simulink model "AcasXuClosedLoop_all_comp2017b"  (Simulink 9.0)
settings: Solver=VariableStepAuto  StartTime=0.0  StopTime=20  RelTol=1e-3
libraries: neural x14040, simulink x2
(part 24 of 534)

== system AcasXuClosedLoop_all_comp2017b/networks/Networks_m_5/AcasXu_5_5/Layer 3 ==
  "a{2} " : Inport  PortDimensions=50
      mask Display: e = 0.06;c1 = [120 230 180]/255;c2 = ([120 230 180]+0)/(256+128);c3 = ([120 230 180]+128)/(256+128);patch([0 1 1 0],[0 0 1 1],c1);patch([0 1 1 1-e 1-e 0+e],[0 0 1 1-e 0+e 0+e],c2);patch([0 0 1 1-e 0+e 0+e],[0 1 1 1-e 1-e 0+e],c3);plot([0 1 1 0 0],[0 0 1 1 0]);
  Delays 1 : Subsystem
      mask Display: e = 0.06;c1 = [120 150 230]/255;c2 = ([120 150 230]+0)/383;c3 = ([120 150 230]+128)/383;patch([0 1 1 0],[0 0 1 1],c1);patch([0 1 1 1-e 1-e 0+e],[0 0 1 1-e 0+e 0+e],c2);patch([0 0 1 1-e 0+e 0+e],[0 1 1 1-e 1-e 0+e],c3);plot([0 1 1 0 0],[0 0 1 1 0]);disp('0');
  "LW{3,2}" : Subsystem
      mask Display: e = 0.06;c1 = [120 150 230]/255;c2 = ([120 150 230]+0)/383;c3 = ([120 150 230]+128)/383;patch([0 1 1 0],[0 0 1 1],c1);patch([0 1 1 1-e 1-e 0+e],[0 0 1 1-e 0+e 0+e],c2);patch([0 0 1 1-e 0+e 0+e],[0 1 1 1-e 1-e 0+e],c3);plot([0 1 1 0 0],[0 0 1 1 0]);disp('W');
  "b{3}" : Constant  Value=[0.7179569999999999563300434601842425763607025146484375;0.73998500000000000387245790989254601299762725830078125;0.450612999999999985778487143761594779789447784423828125;-0.284893999999999980587972459034062922000885009765625;-0.6379829999999999667892325305729173123836517333984375;-0.0190351999999999986490362147151245153509080410003662109375;-0.7305260000000000086828322309884242713451385498046875;-0.134921000000000013141487897883052937686443328857421875;-1.0406800000000000494537744089029729366302490234375;-0.497483999999999981778131541432230733335018157958984375;-1.8671699999999999963762320476234890520572662353515625;-0.431711000000000011400658195270807482302188873291015625;-1.249049999999999993605115378159098327159881591796875;-0.52125699999999997036326249144622124731540679931640625;-0.38881500000000002170708057747106067836284637451171875;-0.230169000000000012473577726268558762967586517333984375;0.2886290000000000244284592554322443902492523193359375;1.158020000000000049311665861750952899456024169921875;0.62621400000000004837374945054762065410614013671875;-0.435367999999999977234210746246390044689178466796875;-0.09715310000000000612008221878568292595446109771728515625;-1.7334400000000000918731757337809540331363677978515625;-0.032423699999999999743494072390603832900524139404296875;0.6112830000000000207904804483405314385890960693359375;0.0296526000000000011791456699938862584531307220458984375;0.806359000000000047947423809091560542583465576171875;0.89186799999999999410960072054876945912837982177734375;-0.352733000000000018747670083030243404209613800048828125;0.77193500000000003780087354243732988834381103515625;0.06677470000000000627249363560622441582381725311279296875;-0.08103789999999999604796130370232276618480682373046875;-0.39701100000000000278532752417959272861480712890625;-0.5575200000000000155608859131461940705776214599609375;-0.00284884999999999999342747969421907328069210052490234375;0.16597600000000001241318159372895024716854095458984375;-0.289582999999999979312548248344683088362216949462890625;-0.346407999999999993701038647486711852252483367919921875;-0.174549000000000009702461056804168038070201873779296875;-0.771900000000000030553337637684307992458343505859375;0.35227799999999998004085455249878577888011932373046875;-0.704188999999999953871565594454295933246612548828125;-0.73722100000000001518429826319334097206592559814453125;-0.037192599999999999271782513687867322005331516265869140625;0.902943000000000051130655265296809375286102294921875;-0.25704300000000002146549604731262661516666412353515625;1.3462799999999999212008106042048893868923187255859375;-0.07558220000000000216022755239464459009468555450439453125;-0.04270920000000000271711542154662311077117919921875;-1.5134700000000000930100441109971143305301666259765625;0.58542000000000005144329406903125345706939697265625]
      mask Display: e = 0.06;c1 = [120 150 230]/255;c2 = ([120 150 230]+0)/383;c3 = ([120 150 230]+128)/383;patch([0 1 1 0],[0 0 1 1],c1);patch([0 1 1 1-e 1-e 0+e],[0 0 1 1-e 0+e 0+e],c2);patch([0 0 1 1-e 0+e 0+e],[0 1 1 1-e 1-e 0+e],c3);plot([0 1 1 0 0],[0 0 1 1 0]);disp('b');
  netsum : Sum  Inputs=2  SaturateOnIntegerOverflow=on  InputSameDT=on  OutDataTypeStr=Inherit: Same as first input
      mask Display: e = 0.06;c1 = [120 150 230]/255;c2 = ([120 150 230]+0)/383;c3 = ([120 150 230]+128)/383;patch([0 1 1 0],[0 0 1 1],c1);patch([0 1 1 1-e 1-e 0+e],[0 0 1 1-e 0+e 0+e],c2);patch([0 0 1 1-e 0+e 0+e],[0 1 1 1-e 1-e 0+e],c3);plot([0 1 1 0 0],[0 0 1 1 0]);disp('+');
  poslin : neural/Transfer Functions/poslin
      settings: SourceProductName=Deep Learning Toolbox  SourceProductBaseCode=NN
  "a{3}" : Outport  InitialOutput=[0;0;0;0;0;0;0;0;0;0;0;0;0;0;0;0;0;0;0;0;0;0;0;0;0;0;0;0;0;0;0;0;0;0;0;0;0;0;0;0;0;0;0;0;0;0;0;0;0;0]
      mask Display: e = 0.06;c1 = [120 230 180]/255;c2 = ([120 230 180]+0)/(256+128);c3 = ([120 230 180]+128)/(256+128);patch([0 1 1 0],[0 0 1 1],c1);patch([0 1 1 1-e 1-e 0+e],[0 0 1 1-e 0+e 0+e],c2);patch([0 0 1 1-e 0+e 0+e],[0 1 1 1-e 1-e 0+e],c3);plot([0 1 1 0 0],[0 0 1 1 0]);
  wires:
  poslin/1 -> "a{3}"/1
  netsum/1 -> poslin/1
  "b{3}"/1 -> netsum/2
  "LW{3,2}"/1("lz{3,2}") -> netsum/1
  Delays 1/1("pd{3,2}") -> "LW{3,2}"/1("ad{3,2}")
  "a{2} "/1 -> Delays 1/1("p{3}")

== system AcasXuClosedLoop_all_comp2017b/networks/Networks_m_5/AcasXu_5_5/Layer 4 ==
  "a{3} " : Inport  PortDimensions=50
      mask Display: e = 0.06;c1 = [120 230 180]/255;c2 = ([120 230 180]+0)/(256+128);c3 = ([120 230 180]+128)/(256+128);patch([0 1 1 0],[0 0 1 1],c1);patch([0 1 1 1-e 1-e 0+e],[0 0 1 1-e 0+e 0+e],c2);patch([0 0 1 1-e 0+e 0+e],[0 1 1 1-e 1-e 0+e],c3);plot([0 1 1 0 0],[0 0 1 1 0]);
  Delays 1 : Subsystem
      mask Display: e = 0.06;c1 = [120 150 230]/255;c2 = ([120 150 230]+0)/383;c3 = ([120 150 230]+128)/383;patch([0 1 1 0],[0 0 1 1],c1);patch([0 1 1 1-e 1-e 0+e],[0 0 1 1-e 0+e 0+e],c2);patch([0 0 1 1-e 0+e 0+e],[0 1 1 1-e 1-e 0+e],c3);plot([0 1 1 0 0],[0 0 1 1 0]);disp('0');
  "LW{4,3}" : Subsystem
      mask Display: e = 0.06;c1 = [120 150 230]/255;c2 = ([120 150 230]+0)/383;c3 = ([120 150 230]+128)/383;patch([0 1 1 0],[0 0 1 1],c1);patch([0 1 1 1-e 1-e 0+e],[0 0 1 1-e 0+e 0+e],c2);patch([0 0 1 1-e 0+e 0+e],[0 1 1 1-e 1-e 0+e],c3);plot([0 1 1 0 0],[0 0 1 1 0]);disp('W');
  "b{4}" : Constant  Value=[0.86561399999999999455013721672003157436847686767578125;-0.84666900000000000492406115881749428808689117431640625;0.0750065000000000037250202922223252244293689727783203125;0.283154999999999990034638130964594893157482147216796875;-4.12460999999999966547648000414483249187469482421875;0.082238699999999997913846527808345854282379150390625;-0.9694540000000000379287712348741479218006134033203125;0.687108999999999969787722875480540096759796142578125;0.515031000000000016569856597925536334514617919921875;-1.0304500000000000881072992342524230480194091796875;-0.038258699999999999652455784371340996585786342620849609375;-1.065770000000000106155084722558967769145965576171875;-2.813629999999999853343979339115321636199951171875;-1.8924099999999999255351212923415005207061767578125;-0.7152680000000000148929757415316998958587646484375;1.711580000000000101323394119390286505222320556640625;-0.619380000000000041637804315541870892047882080078125;-0.5122079999999999966320274324971251189708709716796875;-0.0881904000000000021231016944511793553829193115234375;-0.92888999999999999346300683100707828998565673828125;-0.01584549999999999847499765337488497607409954071044921875;0.50446100000000004826006261282600462436676025390625;-0.042949099999999997001598472934347228147089481353759765625;0.050072899999999996578647909473147592507302761077880859375;-0.75351299999999998835420456089195795357227325439453125;0.62020200000000003104361212535877712070941925048828125;0.686549999999999993605115378159098327159881591796875;2.18803999999999998493649400188587605953216552734375;0.320101000000000024403590259680640883743762969970703125;-2.126560000000000005826450433232821524143218994140625;-2.233659999999999978825826474349014461040496826171875;-0.484528000000000014235723710953607223927974700927734375;0.6396100000000000118660636871936731040477752685546875;1.960879999999999956372676024329848587512969970703125;2.0860500000000001818989403545856475830078125;-2.430330000000000101323394119390286505222320556640625;-1.0909299999999999553068619206896983087062835693359375;-2.556340000000000056701310313655994832515716552734375;-0.1785799999999999887467794223994133062660694122314453125;-0.57670600000000005191935770199052058160305023193359375;4.5265500000000002955857780762016773223876953125;-1.1713299999999999823074858795735053718090057373046875;0.133823999999999998511412968582590110599994659423828125;0.18771999999999999797495320308371447026729583740234375;-0.2106599999999999861532984368750476278364658355712890625;2.045310000000000183462134373257867991924285888671875;-0.72368299999999996519051137511269189417362213134765625;-2.34640000000000004121147867408581078052520751953125;0.553835999999999994969357430818490684032440185546875;-4.1325599999999997891109160264022648334503173828125]
      mask Display: e = 0.06;c1 = [120 150 230]/255;c2 = ([120 150 230]+0)/383;c3 = ([120 150 230]+128)/383;patch([0 1 1 0],[0 0 1 1],c1);patch([0 1 1 1-e 1-e 0+e],[0 0 1 1-e 0+e 0+e],c2);patch([0 0 1 1-e 0+e 0+e],[0 1 1 1-e 1-e 0+e],c3);plot([0 1 1 0 0],[0 0 1 1 0]);disp('b');
  netsum : Sum  Inputs=2  SaturateOnIntegerOverflow=on  InputSameDT=on  OutDataTypeStr=Inherit: Same as first input
      mask Display: e = 0.06;c1 = [120 150 230]/255;c2 = ([120 150 230]+0)/383;c3 = ([120 150 230]+128)/383;patch([0 1 1 0],[0 0 1 1],c1);patch([0 1 1 1-e 1-e 0+e],[0 0 1 1-e 0+e 0+e],c2);patch([0 0 1 1-e 0+e 0+e],[0 1 1 1-e 1-e 0+e],c3);plot([0 1 1 0 0],[0 0 1 1 0]);disp('+');
  poslin : neural/Transfer Functions/poslin
      settings: SourceProductName=Deep Learning Toolbox  SourceProductBaseCode=NN
  "a{4}" : Outport  InitialOutput=[0;0;0;0;0;0;0;0;0;0;0;0;0;0;0;0;0;0;0;0;0;0;0;0;0;0;0;0;0;0;0;0;0;0;0;0;0;0;0;0;0;0;0;0;0;0;0;0;0;0]
      mask Display: e = 0.06;c1 = [120 230 180]/255;c2 = ([120 230 180]+0)/(256+128);c3 = ([120 230 180]+128)/(256+128);patch([0 1 1 0],[0 0 1 1],c1);patch([0 1 1 1-e 1-e 0+e],[0 0 1 1-e 0+e 0+e],c2);patch([0 0 1 1-e 0+e 0+e],[0 1 1 1-e 1-e 0+e],c3);plot([0 1 1 0 0],[0 0 1 1 0]);
  wires:
  poslin/1 -> "a{4}"/1
  netsum/1 -> poslin/1
  "b{4}"/1 -> netsum/2
  "LW{4,3}"/1("lz{4,3}") -> netsum/1
  Delays 1/1("pd{4,3}") -> "LW{4,3}"/1("ad{4,3}")
  "a{3} "/1 -> Delays 1/1("p{4}")

== system AcasXuClosedLoop_all_comp2017b/networks/Networks_m_5/AcasXu_5_5/Layer 5 ==
  "a{4} " : Inport  PortDimensions=50
      mask Display: e = 0.06;c1 = [120 230 180]/255;c2 = ([120 230 180]+0)/(256+128);c3 = ([120 230 180]+128)/(256+128);patch([0 1 1 0],[0 0 1 1],c1);patch([0 1 1 1-e 1-e 0+e],[0 0 1 1-e 0+e 0+e],c2);patch([0 0 1 1-e 0+e 0+e],[0 1 1 1-e 1-e 0+e],c3);plot([0 1 1 0 0],[0 0 1 1 0]);
  Delays 1 : Subsystem
      mask Display: e = 0.06;c1 = [120 150 230]/255;c2 = ([120 150 230]+0)/383;c3 = ([120 150 230]+128)/383;patch([0 1 1 0],[0 0 1 1],c1);patch([0 1 1 1-e 1-e 0+e],[0 0 1 1-e 0+e 0+e],c2);patch([0 0 1 1-e 0+e 0+e],[0 1 1 1-e 1-e 0+e],c3);plot([0 1 1 0 0],[0 0 1 1 0]);disp('0');
  "LW{5,4}" : Subsystem
      mask Display: e = 0.06;c1 = [120 150 230]/255;c2 = ([120 150 230]+0)/383;c3 = ([120 150 230]+128)/383;patch([0 1 1 0],[0 0 1 1],c1);patch([0 1 1 1-e 1-e 0+e],[0 0 1 1-e 0+e 0+e],c2);patch([0 0 1 1-e 0+e 0+e],[0 1 1 1-e 1-e 0+e],c3);plot([0 1 1 0 0],[0 0 1 1 0]);disp('W');
  "b{5}" : Constant  Value=[-0.06693159999999999387743088163915672339498996734619140625;1.0652999999999999136690576051478274166584014892578125;0.58934399999999997898925130357383750379085540771484375;-1.117669999999999941309170026215724647045135498046875;1.2888800000000000256505927609396167099475860595703125;-0.061067499999999996784794120685546658933162689208984375;2.960679999999999978399500832892954349517822265625;-1.6771000000000000351718654201249592006206512451171875;0.0221778000000000009850342763684238889254629611968994140625;3.13361000000000000653699316899292171001434326171875;1.882600000000000051159076974727213382720947265625;0.4156349999999999766941982670687139034271240234375;0.37906899999999998929212097209529019892215728759765625;5.43222000000000004860112312599085271358489990234375;-2.279189999999999827196006663143634796142578125;1.601599999999999912603243501507677137851715087890625;1.5714099999999999734967559561482630670070648193359375;1.9408600000000000296296320811961777508258819580078125;-1.30055999999999993832489053602330386638641357421875;0.055389899999999998858601912843369063921272754669189453125;-0.4228870000000000128892452266882173717021942138671875;-4.1825799999999997424993125605396926403045654296875;-1.2646100000000000118660636871936731040477752685546875;-0.41976299999999999723598875789321027696132659912109375;2.37666000000000021685764295398257672786712646484375;-0.165416000000000007474909580196253955364227294921875;0.969813999999999953871565594454295933246612548828125;1.6141600000000000392219590139575302600860595703125;-1.0070799999999999752020585219725035130977630615234375;-0.333878999999999981351805899976170621812343597412109375;-4.59891999999999967485564411617815494537353515625;-2.1183800000000001517719283583573997020721435546875;0.1611499999999999876987288871532655321061611175537109375;-0.0243379999999999985238474664583918638527393341064453125;0.303306000000000020033752434756024740636348724365234375;-0.376114999999999977120523908524774014949798583984375;2.1257000000000001449507180950604379177093505859375;1.3156399999999999206323764155968092381954193115234375;1.0355399999999999050004362288746051490306854248046875;-0.427047000000000009922729304889799095690250396728515625;0.298074000000000005616840326183591969311237335205078125;2.235170000000000101181285572238266468048095703125;0.89289600000000002300026835655444301664829254150390625;0.56845500000000004359179683888214640319347381591796875;3.46098000000000016740386854507960379123687744140625;-2.865369999999999972573050399660132825374603271484375;-1.1227000000000000312638803734444081783294677734375;-4.03671000000000024243718144134618341922760009765625;-1.3588899999999999312905174519983120262622833251953125;1.3272299999999999098321268320432864129543304443359375]
      mask Display: e = 0.06;c1 = [120 150 230]/255;c2 = ([120 150 230]+0)/383;c3 = ([120 150 230]+128)/383;patch([0 1 1 0],[0 0 1 1],c1);patch([0 1 1 1-e 1-e 0+e],[0 0 1 1-e 0+e 0+e],c2);patch([0 0 1 1-e 0+e 0+e],[0 1 1 1-e 1-e 0+e],c3);plot([0 1 1 0 0],[0 0 1 1 0]);disp('b');
  netsum : Sum  Inputs=2  SaturateOnIntegerOverflow=on  InputSameDT=on  OutDataTypeStr=Inherit: Same as first input
      mask Display: e = 0.06;c1 = [120 150 230]/255;c2 = ([120 150 230]+0)/383;c3 = ([120 150 230]+128)/383;patch([0 1 1 0],[0 0 1 1],c1);patch([0 1 1 1-e 1-e 0+e],[0 0 1 1-e 0+e 0+e],c2);patch([0 0 1 1-e 0+e 0+e],[0 1 1 1-e 1-e 0+e],c3);plot([0 1 1 0 0],[0 0 1 1 0]);disp('+');
  poslin : neural/Transfer Functions/poslin
      settings: SourceProductName=Deep Learning Toolbox  SourceProductBaseCode=NN
  "a{5}" : Outport  InitialOutput=[0;0;0;0;0;0;0;0;0;0;0;0;0;0;0;0;0;0;0;0;0;0;0;0;0;0;0;0;0;0;0;0;0;0;0;0;0;0;0;0;0;0;0;0;0;0;0;0;0;0]
      mask Display: e = 0.06;c1 = [120 230 180]/255;c2 = ([120 230 180]+0)/(256+128);c3 = ([120 230 180]+128)/(256+128);patch([0 1 1 0],[0 0 1 1],c1);patch([0 1 1 1-e 1-e 0+e],[0 0 1 1-e 0+e 0+e],c2);patch([0 0 1 1-e 0+e 0+e],[0 1 1 1-e 1-e 0+e],c3);plot([0 1 1 0 0],[0 0 1 1 0]);
  wires:
  poslin/1 -> "a{5}"/1
  netsum/1 -> poslin/1
  "b{5}"/1 -> netsum/2
  "LW{5,4}"/1("lz{5,4}") -> netsum/1
  Delays 1/1("pd{5,4}") -> "LW{5,4}"/1("ad{5,4}")
  "a{4} "/1 -> Delays 1/1("p{5}")

== system AcasXuClosedLoop_all_comp2017b/networks/Networks_m_5/AcasXu_5_5/Layer 6 ==
  "a{5} " : Inport  PortDimensions=50
      mask Display: e = 0.06;c1 = [120 230 180]/255;c2 = ([120 230 180]+0)/(256+128);c3 = ([120 230 180]+128)/(256+128);patch([0 1 1 0],[0 0 1 1],c1);patch([0 1 1 1-e 1-e 0+e],[0 0 1 1-e 0+e 0+e],c2);patch([0 0 1 1-e 0+e 0+e],[0 1 1 1-e 1-e 0+e],c3);plot([0 1 1 0 0],[0 0 1 1 0]);
  Delays 1 : Subsystem
      mask Display: e = 0.06;c1 = [120 150 230]/255;c2 = ([120 150 230]+0)/383;c3 = ([120 150 230]+128)/383;patch([0 1 1 0],[0 0 1 1],c1);patch([0 1 1 1-e 1-e 0+e],[0 0 1 1-e 0+e 0+e],c2);patch([0 0 1 1-e 0+e 0+e],[0 1 1 1-e 1-e 0+e],c3);plot([0 1 1 0 0],[0 0 1 1 0]);disp('0');
  "LW{6,5}" : Subsystem
      mask Display: e = 0.06;c1 = [120 150 230]/255;c2 = ([120 150 230]+0)/383;c3 = ([120 150 230]+128)/383;patch([0 1 1 0],[0 0 1 1],c1);patch([0 1 1 1-e 1-e 0+e],[0 0 1 1-e 0+e 0+e],c2);patch([0 0 1 1-e 0+e 0+e],[0 1 1 1-e 1-e 0+e],c3);plot([0 1 1 0 0],[0 0 1 1 0]);disp('W');
  "b{6}" : Constant  Value=[0.3363519999999999843254272491321898996829986572265625;-0.496522999999999992137844628814491443336009979248046875;0.1646620000000000028084201630917959846556186676025390625;0.78365899999999999447908294314402155578136444091796875;-2.68461000000000016285639503621496260166168212890625;1.614780000000000104165565062430687248706817626953125;-2.99429999999999996163069226895458996295928955078125;-1.8797200000000000574829073229921050369739532470703125;0.212693999999999994177102280445978976786136627197265625;0.051251100000000000822542034484285977669060230255126953125;0.22147600000000000619593265582807362079620361328125;0.8871809999999999973852027324028313159942626953125;0.58963200000000004497024974625674076378345489501953125;0.31559999999999999165112285481882281601428985595703125;-1.38220000000000009521272659185342490673065185546875;-0.5588020000000000209183781407773494720458984375;0.81517600000000001170263885796885006129741668701171875;0.18274299999999998878053020234801806509494781494140625;1.0703300000000000036237679523765109479427337646484375;0.765068999999999999062083588796667754650115966796875;0.320755999999999985572429750391165725886821746826171875;0.9126250000000000195399252334027551114559173583984375;-1.621329999999999937898564894567243754863739013671875;-3.348730000000000206483719011885114014148712158203125;0.051210600000000001952304984342845273204147815704345703125;1.5204599999999999226929503493010997772216796875;0.69752599999999997937294438088429160416126251220703125;-2.27393000000000000682121026329696178436279296875;-1.1254200000000000869704308570362627506256103515625;-0.47547200000000000574829073229921050369739532470703125;2.781120000000000036521896618069149553775787353515625;1.0138700000000000489563944938709028065204620361328125;-1.511670000000000069206862463033758103847503662109375;-2.94512999999999980360598783590830862522125244140625;-2.171170000000000044337866711430251598358154296875;0.54944300000000001471534005759167484939098358154296875;1.1577599999999999003108541728579439222812652587890625;0.9550929999999999697735120207653380930423736572265625;0.9718970000000000108997255665599368512630462646484375;0.415206000000000019500845382935949601233005523681640625;1.383739999999999970015096550923772156238555908203125;0.371655000000000013127277043167850933969020843505859375;0.4622370000000000089812601800076663494110107421875;3.474660000000000081854523159563541412353515625;3.068989999999999884749968259711749851703643798828125;-0.871264000000000038426151149906218051910400390625;-1.2139200000000001100630697692395187914371490478515625;-0.94466799999999995218757931070285849273204803466796875;1.2405699999999999505462255910970270633697509765625;-0.7336899999999999533173422605614177882671356201171875]
      mask Display: e = 0.06;c1 = [120 150 230]/255;c2 = ([120 150 230]+0)/383;c3 = ([120 150 230]+128)/383;patch([0 1 1 0],[0 0 1 1],c1);patch([0 1 1 1-e 1-e 0+e],[0 0 1 1-e 0+e 0+e],c2);patch([0 0 1 1-e 0+e 0+e],[0 1 1 1-e 1-e 0+e],c3);plot([0 1 1 0 0],[0 0 1 1 0]);disp('b');
  netsum : Sum  Inputs=2  SaturateOnIntegerOverflow=on  InputSameDT=on  OutDataTypeStr=Inherit: Same as first input
      mask Display: e = 0.06;c1 = [120 150 230]/255;c2 = ([120 150 230]+0)/383;c3 = ([120 150 230]+128)/383;patch([0 1 1 0],[0 0 1 1],c1);patch([0 1 1 1-e 1-e 0+e],[0 0 1 1-e 0+e 0+e],c2);patch([0 0 1 1-e 0+e 0+e],[0 1 1 1-e 1-e 0+e],c3);plot([0 1 1 0 0],[0 0 1 1 0]);disp('+');
  poslin : neural/Transfer Functions/poslin
      settings: SourceProductName=Deep Learning Toolbox  SourceProductBaseCode=NN
  "a{6}" : Outport  InitialOutput=[0;0;0;0;0;0;0;0;0;0;0;0;0;0;0;0;0;0;0;0;0;0;0;0;0;0;0;0;0;0;0;0;0;0;0;0;0;0;0;0;0;0;0;0;0;0;0;0;0;0]
      mask Display: e = 0.06;c1 = [120 230 180]/255;c2 = ([120 230 180]+0)/(256+128);c3 = ([120 230 180]+128)/(256+128);patch([0 1 1 0],[0 0 1 1],c1);patch([0 1 1 1-e 1-e 0+e],[0 0 1 1-e 0+e 0+e],c2);patch([0 0 1 1-e 0+e 0+e],[0 1 1 1-e 1-e 0+e],c3);plot([0 1 1 0 0],[0 0 1 1 0]);
  wires:
  poslin/1 -> "a{6}"/1
  netsum/1 -> poslin/1
  "b{6}"/1 -> netsum/2
  "LW{6,5}"/1("lz{6,5}") -> netsum/1
  Delays 1/1("pd{6,5}") -> "LW{6,5}"/1("ad{6,5}")
  "a{5} "/1 -> Delays 1/1("p{6}")

== system AcasXuClosedLoop_all_comp2017b/networks/Networks_m_5/AcasXu_5_5/Layer 7 ==
  "a{6} " : Inport  PortDimensions=50
      mask Display: e = 0.06;c1 = [120 230 180]/255;c2 = ([120 230 180]+0)/(256+128);c3 = ([120 230 180]+128)/(256+128);patch([0 1 1 0],[0 0 1 1],c1);patch([0 1 1 1-e 1-e 0+e],[0 0 1 1-e 0+e 0+e],c2);patch([0 0 1 1-e 0+e 0+e],[0 1 1 1-e 1-e 0+e],c3);plot([0 1 1 0 0],[0 0 1 1 0]);
  Delays 1 : Subsystem
      mask Display: e = 0.06;c1 = [120 150 230]/255;c2 = ([120 150 230]+0)/383;c3 = ([120 150 230]+128)/383;patch([0 1 1 0],[0 0 1 1],c1);patch([0 1 1 1-e 1-e 0+e],[0 0 1 1-e 0+e 0+e],c2);patch([0 0 1 1-e 0+e 0+e],[0 1 1 1-e 1-e 0+e],c3);plot([0 1 1 0 0],[0 0 1 1 0]);disp('0');
  "LW{7,6}" : Subsystem
      mask Display: e = 0.06;c1 = [120 150 230]/255;c2 = ([120 150 230]+0)/383;c3 = ([120 150 230]+128)/383;patch([0 1 1 0],[0 0 1 1],c1);patch([0 1 1 1-e 1-e 0+e],[0 0 1 1-e 0+e 0+e],c2);patch([0 0 1 1-e 0+e 0+e],[0 1 1 1-e 1-e 0+e],c3);plot([0 1 1 0 0],[0 0 1 1 0]);disp('W');
  "b{7}" : Constant  Value=[-0.021560400000000000397815114183686091564595699310302734375;0.0182964000000000008572254017735758679918944835662841796875;-0.0184361000000000004039879542006019619293510913848876953125;0.0185537999999999987654764055378109333105385303497314453125;-0.0175339000000000015677681375336760538630187511444091796875]
      mask Display: e = 0.06;c1 = [120 150 230]/255;c2 = ([120 150 230]+0)/383;c3 = ([120 150 230]+128)/383;patch([0 1 1 0],[0 0 1 1],c1);patch([0 1 1 1-e 1-e 0+e],[0 0 1 1-e 0+e 0+e],c2);patch([0 0 1 1-e 0+e 0+e],[0 1 1 1-e 1-e 0+e],c3);plot([0 1 1 0 0],[0 0 1 1 0]);disp('b');
  netsum : Sum  Inputs=2  SaturateOnIntegerOverflow=on  InputSameDT=on  OutDataTypeStr=Inherit: Same as first input
      mask Display: e = 0.06;c1 = [120 150 230]/255;c2 = ([120 150 230]+0)/383;c3 = ([120 150 230]+128)/383;patch([0 1 1 0],[0 0 1 1],c1);patch([0 1 1 1-e 1-e 0+e],[0 0 1 1-e 0+e 0+e],c2);patch([0 0 1 1-e 0+e 0+e],[0 1 1 1-e 1-e 0+e],c3);plot([0 1 1 0 0],[0 0 1 1 0]);disp('+');
  purelin : neural/Transfer Functions/purelin
      settings: SourceProductName=Deep Learning Toolbox
  "a{7}" : Outport  InitialOutput=[0;0;0;0;0]
      mask Display: e = 0.06;c1 = [120 230 180]/255;c2 = ([120 230 180]+0)/(256+128);c3 = ([120 230 180]+128)/(256+128);patch([0 1 1 0],[0 0 1 1],c1);patch([0 1 1 1-e 1-e 0+e],[0 0 1 1-e 0+e 0+e],c2);patch([0 0 1 1-e 0+e 0+e],[0 1 1 1-e 1-e 0+e],c3);plot([0 1 1 0 0],[0 0 1 1 0]);
  wires:
  purelin/1 -> "a{7}"/1
  netsum/1 -> purelin/1
  "b{7}"/1 -> netsum/2
  "LW{7,6}"/1("lz{7,6}") -> netsum/1
  Delays 1/1("pd{7,6}") -> "LW{7,6}"/1("ad{7,6}")
  "a{6} "/1 -> Delays 1/1("p{7}")

== system AcasXuClosedLoop_all_comp2017b/networks/Networks_m_5/AcasXu_5_5/Process Input 1 ==
  x : Inport  PortDimensions=5
      mask Display: e = 0.06;c1 = [120 230 180]/255;c2 = ([120 230 180]+0)/(256+128);c3 = ([120 230 180]+128)/(256+128);patch([0 1 1 0],[0 0 1 1],c1);patch([0 1 1 1-e 1-e 0+e],[0 0 1 1-e 0+e 0+e],c2);patch([0 0 1 1-e 0+e 0+e],[0 1 1 1-e 1-e 0+e],c3);plot([0 1 1 0 0],[0 0 1 1 0]);
  p : Outport  PortDimensions=5
      mask Display: e = 0.06;c1 = [120 230 180]/255;c2 = ([120 230 180]+0)/(256+128);c3 = ([120 230 180]+128)/(256+128);patch([0 1 1 0],[0 0 1 1],c1);patch([0 1 1 1-e 1-e 0+e],[0 0 1 1-e 0+e 0+e],c2);patch([0 0 1 1-e 0+e 0+e],[0 1 1 1-e 1-e 0+e],c3);plot([0 1 1 0 0],[0 0 1 1 0]);
  wires:
  x/1 -> p/1

== system AcasXuClosedLoop_all_comp2017b/networks/Networks_m_5/AcasXu_5_5/Process Output 1 ==
  a : Inport  PortDimensions=5
      mask Display: e = 0.06;c1 = [120 230 180]/255;c2 = ([120 230 180]+0)/(256+128);c3 = ([120 230 180]+128)/(256+128);patch([0 1 1 0],[0 0 1 1],c1);patch([0 1 1 1-e 1-e 0+e],[0 0 1 1-e 0+e 0+e],c2);patch([0 0 1 1-e 0+e 0+e],[0 1 1 1-e 1-e 0+e],c3);plot([0 1 1 0 0],[0 0 1 1 0]);
  y : Outport  PortDimensions=5
      mask Display: e = 0.06;c1 = [120 230 180]/255;c2 = ([120 230 180]+0)/(256+128);c3 = ([120 230 180]+128)/(256+128);patch([0 1 1 0],[0 0 1 1],c1);patch([0 1 1 1-e 1-e 0+e],[0 0 1 1-e 0+e 0+e],c2);patch([0 0 1 1-e 0+e 0+e],[0 1 1 1-e 1-e 0+e],c3);plot([0 1 1 0 0],[0 0 1 1 0]);
  wires:
  a/1 -> y/1

== system AcasXuClosedLoop_all_comp2017b/networks/Networks_m_6/AcasXu_1_6/Layer 1 ==
  "p{1}" : Inport  PortDimensions=5
      mask Display: e = 0.06;c1 = [120 230 180]/255;c2 = ([120 230 180]+0)/(256+128);c3 = ([120 230 180]+128)/(256+128);patch([0 1 1 0],[0 0 1 1],c1);patch([0 1 1 1-e 1-e 0+e],[0 0 1 1-e 0+e 0+e],c2);patch([0 0 1 1-e 0+e 0+e],[0 1 1 1-e 1-e 0+e],c3);plot([0 1 1 0 0],[0 0 1 1 0]);
  Delays 1 : Subsystem
      mask Display: e = 0.06;c1 = [120 150 230]/255;c2 = ([120 150 230]+0)/383;c3 = ([120 150 230]+128)/383;patch([0 1 1 0],[0 0 1 1],c1);patch([0 1 1 1-e 1-e 0+e],[0 0 1 1-e 0+e 0+e],c2);patch([0 0 1 1-e 0+e 0+e],[0 1 1 1-e 1-e 0+e],c3);plot([0 1 1 0 0],[0 0 1 1 0]);disp('0');
  "IW{1,1}" : Subsystem
      mask Display: e = 0.06;c1 = [120 150 230]/255;c2 = ([120 150 230]+0)/383;c3 = ([120 150 230]+128)/383;patch([0 1 1 0],[0 0 1 1],c1);patch([0 1 1 1-e 1-e 0+e],[0 0 1 1-e 0+e 0+e],c2);patch([0 0 1 1-e 0+e 0+e],[0 1 1 1-e 1-e 0+e],c3);plot([0 1 1 0 0],[0 0 1 1 0]);disp('W');
  "b{1}" : Constant  Value=[0.0294443999999999990457855147951704566366970539093017578125;-0.01544360000000000003594902153736256877891719341278076171875;0.001673280000000000079796169671908501186408102512359619140625;-0.0172477000000000012247536318454876891337335109710693359375;0.0305429000000000012982059871546880458481609821319580078125;0.0805957999999999952223106447490863502025604248046875;0.09026230000000000364135388508657342754304409027099609375;0.0763289000000000050771831183737958781421184539794921875;0.00141074999999999989470922390211171659757383167743682861328125;0.035209999999999998243627175043002353049814701080322265625;-0.322149999999999991917576380728860385715961456298828125;-0.273977999999999999314326259991503320634365081787109375;-0.248472999999999999420907670355518348515033721923828125;0.07278679999999999872262179678727989085018634796142578125;-0.0207039000000000007750688979513142840005457401275634765625;-0.1951920000000000043893777501580188982188701629638671875;0.02087429999999999841175934989223605953156948089599609375;-0.017661599999999999521804738833452574908733367919921875;-0.0224841999999999993253840813167698797769844532012939453125;-0.3178929999999999811421957929269410669803619384765625;0.1110349999999999948130380289512686431407928466796875;-0.087941400000000002901145990108489058911800384521484375;-0.269813000000000025035973294507130049169063568115234375;-0.1811750000000000027089441800853819586336612701416015625;-0.033771099999999998342303797471686266362667083740234375;-0.014546099999999999308197828895572456531226634979248046875;-0.0176908999999999989871657390949621913023293018341064453125;-0.050836300000000000931521526581491343677043914794921875;-0.1903060000000000029363178555286140181124210357666015625;0.032591500000000002412736677115390193648636341094970703125;0.13095000000000001083577672034152783453464508056640625;0.006100689999999999758861779497465249733068048954010009765625;-0.134761999999999992905230783435399644076824188232421875;-0.310597000000000011965539670200087130069732666015625;0.01118700000000000076283424021994505892507731914520263671875;0.11955200000000000548627809848767356015741825103759765625;0.04915629999999999999449329379785922355949878692626953125;0.010336800000000000154809498553731827996671199798583984375;0.06508139999999999758539814820323954336345195770263671875;-0.01163329999999999919690907290714676491916179656982421875;0.10397499999999999797939409518221509642899036407470703125;0.0075597900000000002596056702941496041603386402130126953125;0.2310280000000000111270992420031689107418060302734375;0.2087360000000000048725468104748870246112346649169921875;-0.0208956999999999996908695010233714128844439983367919921875;-0.046587099999999999344613144103277591057121753692626953125;-0.313607000000000024630963935123872943222522735595703125;-0.0311411000000000014187317987079950398765504360198974609375;0.09075560000000000571152014572362531907856464385986328125;-0.030704900000000000248601139674065052531659603118896484375]
      mask Display: e = 0.06;c1 = [120 150 230]/255;c2 = ([120 150 230]+0)/383;c3 = ([120 150 230]+128)/383;patch([0 1 1 0],[0 0 1 1],c1);patch([0 1 1 1-e 1-e 0+e],[0 0 1 1-e 0+e 0+e],c2);patch([0 0 1 1-e 0+e 0+e],[0 1 1 1-e 1-e 0+e],c3);plot([0 1 1 0 0],[0 0 1 1 0]);disp('b');
  netsum : Sum  Inputs=2  SaturateOnIntegerOverflow=on  InputSameDT=on  OutDataTypeStr=Inherit: Same as first input
      mask Display: e = 0.06;c1 = [120 150 230]/255;c2 = ([120 150 230]+0)/383;c3 = ([120 150 230]+128)/383;patch([0 1 1 0],[0 0 1 1],c1);patch([0 1 1 1-e 1-e 0+e],[0 0 1 1-e 0+e 0+e],c2);patch([0 0 1 1-e 0+e 0+e],[0 1 1 1-e 1-e 0+e],c3);plot([0 1 1 0 0],[0 0 1 1 0]);disp('+');
  poslin : neural/Transfer Functions/poslin
      settings: SourceProductName=Deep Learning Toolbox  SourceProductBaseCode=NN
  "a{1}" : Outport  InitialOutput=[0;0;0;0;0;0;0;0;0;0;0;0;0;0;0;0;0;0;0;0;0;0;0;0;0;0;0;0;0;0;0;0;0;0;0;0;0;0;0;0;0;0;0;0;0;0;0;0;0;0]
      mask Display: e = 0.06;c1 = [120 230 180]/255;c2 = ([120 230 180]+0)/(256+128);c3 = ([120 230 180]+128)/(256+128);patch([0 1 1 0],[0 0 1 1],c1);patch([0 1 1 1-e 1-e 0+e],[0 0 1 1-e 0+e 0+e],c2);patch([0 0 1 1-e 0+e 0+e],[0 1 1 1-e 1-e 0+e],c3);plot([0 1 1 0 0],[0 0 1 1 0]);
  wires:
  poslin/1 -> "a{1}"/1
  netsum/1 -> poslin/1
  "b{1}"/1 -> netsum/2
  "IW{1,1}"/1("iz{1,1}") -> netsum/1
  Delays 1/1("pd{1,1}") -> "IW{1,1}"/1("pd{1,1}")
  "p{1}"/1 -> Delays 1/1("p{1}")

== system AcasXuClosedLoop_all_comp2017b/networks/Networks_m_6/AcasXu_1_6/Layer 2 ==
  "a{1} " : Inport  PortDimensions=50
      mask Display: e = 0.06;c1 = [120 230 180]/255;c2 = ([120 230 180]+0)/(256+128);c3 = ([120 230 180]+128)/(256+128);patch([0 1 1 0],[0 0 1 1],c1);patch([0 1 1 1-e 1-e 0+e],[0 0 1 1-e 0+e 0+e],c2);patch([0 0 1 1-e 0+e 0+e],[0 1 1 1-e 1-e 0+e],c3);plot([0 1 1 0 0],[0 0 1 1 0]);
  Delays 1 : Subsystem
      mask Display: e = 0.06;c1 = [120 150 230]/255;c2 = ([120 150 230]+0)/383;c3 = ([120 150 230]+128)/383;patch([0 1 1 0],[0 0 1 1],c1);patch([0 1 1 1-e 1-e 0+e],[0 0 1 1-e 0+e 0+e],c2);patch([0 0 1 1-e 0+e 0+e],[0 1 1 1-e 1-e 0+e],c3);plot([0 1 1 0 0],[0 0 1 1 0]);disp('0');
  "LW{2,1}" : Subsystem
      mask Display: e = 0.06;c1 = [120 150 230]/255;c2 = ([120 150 230]+0)/383;c3 = ([120 150 230]+128)/383;patch([0 1 1 0],[0 0 1 1],c1);patch([0 1 1 1-e 1-e 0+e],[0 0 1 1-e 0+e 0+e],c2);patch([0 0 1 1-e 0+e 0+e],[0 1 1 1-e 1-e 0+e],c3);plot([0 1 1 0 0],[0 0 1 1 0]);disp('W');
  "b{2}" : Constant  Value=[-0.00856845000000000002471356452815598458983004093170166015625;0.095637899999999997913135985072585754096508026123046875;-0.317821999999999993402610698467469774186611175537109375;-0.2409840000000000037605474290103302337229251861572265625;0.0268840000000000016455725670994070242159068584442138671875;0.2270620000000000138218325673733488656580448150634765625;-0.467696999999999973862685465064714662730693817138671875;-0.2259919999999999984385823381671798415482044219970703125;-0.062017700000000001991917741861470858566462993621826171875;-0.331811000000000022591706283492385409772396087646484375;-0.3039789999999999992041921359486877918243408203125;-0.019253499999999999781064019543919130228459835052490234375;0.394789999999999974278352965484373271465301513671875;-0.007517949999999999806388206735618950915522873401641845703125;0.213117000000000000881072992342524230480194091796875;-0.045447200000000000097433172641103737987577915191650390625;0.32258799999999998586730498573160730302333831787109375;-0.2382910000000000028119728767705964855849742889404296875;-0.017601100000000001466471388766876771114766597747802734375;-0.01743039999999999867252853391619282774627208709716796875;-0.27655499999999999527489080719533376395702362060546875;0.09610390000000000598756599856642424128949642181396484375;0.1600499999999999978239628717346931807696819305419921875;-0.184640999999999999570121644865139387547969818115234375;-0.2047849999999999948130380289512686431407928466796875;-0.0177105000000000005477840403500522370450198650360107421875;0.303798999999999985721643724900786764919757843017578125;-0.0854270000000000029327651418498135171830654144287109375;0.11146999999999999964028774002144928090274333953857421875;0.2155140000000000111146647441273671574890613555908203125;-0.01274529999999999933801841933700416120700538158416748046875;-0.01405320000000000006667999485898690181784331798553466796875;0.40151199999999998002664369778358377516269683837890625;-0.003216459999999999978814724244102762895636260509490966796875;-0.0686029999999999973159248156662215478718280792236328125;-0.05707099999999999673239159392323927022516727447509765625;0.1767390000000000072066796974468161351978778839111328125;-0.278629999999999988791188343384419567883014678955078125;-0.01716890000000000082724938010869664140045642852783203125;-0.070935899999999996179411709817941300570964813232421875;-0.01940329999999999832649422160102403722703456878662109375;-0.20918300000000000782307552071870304644107818603515625;-0.005764749999999999909017223131968421512283384799957275390625;0.41727700000000000901678731679567135870456695556640625;-0.28466000000000002412292587905540131032466888427734375;-0.008250369999999999925943683365403558127582073211669921875;-0.134326000000000000955679979597334749996662139892578125;-0.1947979999999999989324095395204494707286357879638671875;-0.513956999999999997186250766390003263950347900390625;-0.3314500000000000223820961764431558549404144287109375]
      mask Display: e = 0.06;c1 = [120 150 230]/255;c2 = ([120 150 230]+0)/383;c3 = ([120 150 230]+128)/383;patch([0 1 1 0],[0 0 1 1],c1);patch([0 1 1 1-e 1-e 0+e],[0 0 1 1-e 0+e 0+e],c2);patch([0 0 1 1-e 0+e 0+e],[0 1 1 1-e 1-e 0+e],c3);plot([0 1 1 0 0],[0 0 1 1 0]);disp('b');
  netsum : Sum  Inputs=2  SaturateOnIntegerOverflow=on  InputSameDT=on  OutDataTypeStr=Inherit: Same as first input
      mask Display: e = 0.06;c1 = [120 150 230]/255;c2 = ([120 150 230]+0)/383;c3 = ([120 150 230]+128)/383;patch([0 1 1 0],[0 0 1 1],c1);patch([0 1 1 1-e 1-e 0+e],[0 0 1 1-e 0+e 0+e],c2);patch([0 0 1 1-e 0+e 0+e],[0 1 1 1-e 1-e 0+e],c3);plot([0 1 1 0 0],[0 0 1 1 0]);disp('+');
  poslin : neural/Transfer Functions/poslin
      settings: SourceProductName=Deep Learning Toolbox  SourceProductBaseCode=NN
  "a{2}" : Outport  InitialOutput=[0;0;0;0;0;0;0;0;0;0;0;0;0;0;0;0;0;0;0;0;0;0;0;0;0;0;0;0;0;0;0;0;0;0;0;0;0;0;0;0;0;0;0;0;0;0;0;0;0;0]
      mask Display: e = 0.06;c1 = [120 230 180]/255;c2 = ([120 230 180]+0)/(256+128);c3 = ([120 230 180]+128)/(256+128);patch([0 1 1 0],[0 0 1 1],c1);patch([0 1 1 1-e 1-e 0+e],[0 0 1 1-e 0+e 0+e],c2);patch([0 0 1 1-e 0+e 0+e],[0 1 1 1-e 1-e 0+e],c3);plot([0 1 1 0 0],[0 0 1 1 0]);
  wires:
  poslin/1 -> "a{2}"/1
  netsum/1 -> poslin/1
  "b{2}"/1 -> netsum/2
  "LW{2,1}"/1("lz{2,1}") -> netsum/1
  Delays 1/1("pd{2,1}") -> "LW{2,1}"/1("ad{2,1}")
  "a{1} "/1 -> Delays 1/1("p{2}")

== system AcasXuClosedLoop_all_comp2017b/networks/Networks_m_6/AcasXu_1_6/Layer 3 ==
  "a{2} " : Inport  PortDimensions=50
      mask Display: e = 0.06;c1 = [120 230 180]/255;c2 = ([120 230 180]+0)/(256+128);c3 = ([120 230 180]+128)/(256+128);patch([0 1 1 0],[0 0 1 1],c1);patch([0 1 1 1-e 1-e 0+e],[0 0 1 1-e 0+e 0+e],c2);patch([0 0 1 1-e 0+e 0+e],[0 1 1 1-e 1-e 0+e],c3);plot([0 1 1 0 0],[0 0 1 1 0]);
  Delays 1 : Subsystem
      mask Display: e = 0.06;c1 = [120 150 230]/255;c2 = ([120 150 230]+0)/383;c3 = ([120 150 230]+128)/383;patch([0 1 1 0],[0 0 1 1],c1);patch([0 1 1 1-e 1-e 0+e],[0 0 1 1-e 0+e 0+e],c2);patch([0 0 1 1-e 0+e 0+e],[0 1 1 1-e 1-e 0+e],c3);plot([0 1 1 0 0],[0 0 1 1 0]);disp('0');
  "LW{3,2}" : Subsystem
      mask Display: e = 0.06;c1 = [120 150 230]/255;c2 = ([120 150 230]+0)/383;c3 = ([120 150 230]+128)/383;patch([0 1 1 0],[0 0 1 1],c1);patch([0 1 1 1-e 1-e 0+e],[0 0 1 1-e 0+e 0+e],c2);patch([0 0 1 1-e 0+e 0+e],[0 1 1 1-e 1-e 0+e],c3);plot([0 1 1 0 0],[0 0 1 1 0]);disp('W');
  "b{3}" : Constant  Value=[-0.29968600000000000793676235844031907618045806884765625;-0.00257513000000000012723599951414144015870988368988037109375;-0.31281199999999997896082959414343349635601043701171875;-0.0166993000000000002547295707699959166347980499267578125;0.43991400000000002723510306168464012444019317626953125;-0.037436999999999998056665617696125991642475128173828125;-0.326448000000000015941026276777847670018672943115234375;-0.195485999999999993104182749448227696120738983154296875;0.05696690000000000086988194425430265255272388458251953125;-0.501916000000000028791191652999259531497955322265625;-0.373765999999999987135623769063386134803295135498046875;0.08520170000000000520845588880547438748180866241455078125;0.27433600000000002427924528092262335121631622314453125;-0.2107390000000000096491703516221605241298675537109375;-0.01074619999999999915563098085158344474621117115020751953125;0.2325230000000000074589223686416517011821269989013671875;-0.42563099999999998157562686174060218036174774169921875;0.0596546000000000020691004465334117412567138671875;-0.047407499999999998252508959240003605373203754425048828125;0.2365629999999999955040408394779660739004611968994140625;-0.20022200000000001107736125049996189773082733154296875;-0.26478699999999999459276978086563758552074432373046875;-0.036452900000000003355449251785103115253150463104248046875;0.018861099999999998699795611400986672379076480865478515625;-0.1027079999999999937454475684717181138694286346435546875;-0.0079570700000000001150812778405452263541519641876220703125;0.334384000000000014551915228366851806640625;0.35779199999999999892708046900224871933460235595703125;0.2344380000000000074944495054296567104756832122802734375;-0.2301580000000000014725998198628076352179050445556640625;0.1045999999999999985345056074947933666408061981201171875;0.375182999999999988727239497166010551154613494873046875;-0.06595779999999999698712116469323518685996532440185546875;-0.11317000000000000670130617663744487799704074859619140625;-0.0450768000000000002014388655879884026944637298583984375;-0.07655530000000000667714772362160147167742252349853515625;0.1458569999999999866613364929435192607343196868896484375;0.1878559999999999952091656041375244967639446258544921875;-0.0155104999999999999815702977912224014289677143096923828125;-0.0193418999999999986771914706196184852160513401031494140625;0.227650999999999992251531466536107473075389862060546875;-0.01282750000000000036026737149086329736746847629547119140625;0.2155229999999999923598892337395227514207363128662109375;-0.2230149999999999910205161768317339010536670684814453125;0.08002399999999999791189253528500557877123355865478515625;0.00810435999999999968912423042866066680289804935455322265625;0.302148000000000027664981416819500736892223358154296875;-0.364742999999999983895548893997329287230968475341796875;-0.2729530000000000011795009413617663085460662841796875;0.0777072000000000040476066942574107088148593902587890625]
      mask Display: e = 0.06;c1 = [120 150 230]/255;c2 = ([120 150 230]+0)/383;c3 = ([120 150 230]+128)/383;patch([0 1 1 0],[0 0 1 1],c1);patch([0 1 1 1-e 1-e 0+e],[0 0 1 1-e 0+e 0+e],c2);patch([0 0 1 1-e 0+e 0+e],[0 1 1 1-e 1-e 0+e],c3);plot([0 1 1 0 0],[0 0 1 1 0]);disp('b');
  netsum : Sum  Inputs=2  SaturateOnIntegerOverflow=on  InputSameDT=on  OutDataTypeStr=Inherit: Same as first input
      mask Display: e = 0.06;c1 = [120 150 230]/255;c2 = ([120 150 230]+0)/383;c3 = ([120 150 230]+128)/383;patch([0 1 1 0],[0 0 1 1],c1);patch([0 1 1 1-e 1-e 0+e],[0 0 1 1-e 0+e 0+e],c2);patch([0 0 1 1-e 0+e 0+e],[0 1 1 1-e 1-e 0+e],c3);plot([0 1 1 0 0],[0 0 1 1 0]);disp('+');
  poslin : neural/Transfer Functions/poslin
      settings: SourceProductName=Deep Learning Toolbox  SourceProductBaseCode=NN
  "a{3}" : Outport  InitialOutput=[0;0;0;0;0;0;0;0;0;0;0;0;0;0;0;0;0;0;0;0;0;0;0;0;0;0;0;0;0;0;0;0;0;0;0;0;0;0;0;0;0;0;0;0;0;0;0;0;0;0]
      mask Display: e = 0.06;c1 = [120 230 180]/255;c2 = ([120 230 180]+0)/(256+128);c3 = ([120 230 180]+128)/(256+128);patch([0 1 1 0],[0 0 1 1],c1);patch([0 1 1 1-e 1-e 0+e],[0 0 1 1-e 0+e 0+e],c2);patch([0 0 1 1-e 0+e 0+e],[0 1 1 1-e 1-e 0+e],c3);plot([0 1 1 0 0],[0 0 1 1 0]);
  wires:
  poslin/1 -> "a{3}"/1
  netsum/1 -> poslin/1
  "b{3}"/1 -> netsum/2
  "LW{3,2}"/1("lz{3,2}") -> netsum/1
  Delays 1/1("pd{3,2}") -> "LW{3,2}"/1("ad{3,2}")
  "a{2} "/1 -> Delays 1/1("p{3}")

== system AcasXuClosedLoop_all_comp2017b/networks/Networks_m_6/AcasXu_1_6/Layer 4 ==
  "a{3} " : Inport  PortDimensions=50
      mask Display: e = 0.06;c1 = [120 230 180]/255;c2 = ([120 230 180]+0)/(256+128);c3 = ([120 230 180]+128)/(256+128);patch([0 1 1 0],[0 0 1 1],c1);patch([0 1 1 1-e 1-e 0+e],[0 0 1 1-e 0+e 0+e],c2);patch([0 0 1 1-e 0+e 0+e],[0 1 1 1-e 1-e 0+e],c3);plot([0 1 1 0 0],[0 0 1 1 0]);
  Delays 1 : Subsystem
      mask Display: e = 0.06;c1 = [120 150 230]/255;c2 = ([120 150 230]+0)/383;c3 = ([120 150 230]+128)/383;patch([0 1 1 0],[0 0 1 1],c1);patch([0 1 1 1-e 1-e 0+e],[0 0 1 1-e 0+e 0+e],c2);patch([0 0 1 1-e 0+e 0+e],[0 1 1 1-e 1-e 0+e],c3);plot([0 1 1 0 0],[0 0 1 1 0]);disp('0');
  "LW{4,3}" : Subsystem
      mask Display: e = 0.06;c1 = [120 150 230]/255;c2 = ([120 150 230]+0)/383;c3 = ([120 150 230]+128)/383;patch([0 1 1 0],[0 0 1 1],c1);patch([0 1 1 1-e 1-e 0+e],[0 0 1 1-e 0+e 0+e],c2);patch([0 0 1 1-e 0+e 0+e],[0 1 1 1-e 1-e 0+e],c3);plot([0 1 1 0 0],[0 0 1 1 0]);disp('W');
  "b{4}" : Constant  Value=[-0.3150399999999999867128508412861265242099761962890625;-0.33053900000000002723510306168464012444019317626953125;-0.1608759999999999912301262838809634558856487274169921875;-0.84662899999999996492050513552385382354259490966796875;-0.3061920000000000197104554899851791560649871826171875;0.169306000000000012040146657454897649586200714111328125;0.356700000000000017053025658242404460906982421875;0.0125304000000000005654587909020847291685640811920166015625;-0.1397879999999999955928586814479785971343517303466796875;0.05681540000000000201207939198866370134055614471435546875;-0.0231085000000000005682121440031551173888146877288818359375;0.0345210999999999990084376122467801906168460845947265625;-0.3423760000000000136566313813091255724430084228515625;-0.1144259999999999999342747969421907328069210052490234375;-0.1942689999999999972413178284114110283553600311279296875;0.09097439999999999693525154498274787329137325286865234375;-0.3126780000000000114823706098832190036773681640625;0.1291420000000000067874594833483570255339145660400390625;-0.07555989999999999928714800034867948852479457855224609375;-0.308035999999999976495246301055885851383209228515625;-0.3872079999999999966320274324971251189708709716796875;0.055553100000000001090771917233723797835409641265869140625;-0.25593900000000002759037442956469021737575531005859375;0.11864500000000000046185277824406512081623077392578125;-0.2105559999999999931663552388272364623844623565673828125;-0.057582500000000001738609256562995142303407192230224609375;-0.220594000000000012295942042328533716499805450439453125;-0.659518999999999966377117743832059204578399658203125;-1.2012300000000000199662508748588152229785919189453125;-0.442616999999999982673415388489956967532634735107421875;0.014038399999999999490096769250158104114234447479248046875;-0.93725000000000002753353101070388220250606536865234375;0.236646999999999996244781641507870517671108245849609375;-0.0066580800000000002480948779748359811492264270782470703125;0.98412800000000000277822209682199172675609588623046875;0.018426299999999999623678803573056939058005809783935546875;-0.1340159999999999962394525709896697662770748138427734375;-0.759994999999999976125764078460633754730224609375;0.02662319999999999964312991096448968164622783660888671875;-0.872870999999999952478901832364499568939208984375;0.192630999999999996674659996642731130123138427734375;0.039846100000000002350208916368501377291977405548095703125;-0.32200499999999998568256387443398125469684600830078125;-1.2349300000000000832045543575077317655086517333984375;0.1667180000000000050786042038453160785138607025146484375;-0.013198200000000000209343653523319517262279987335205078125;-0.1810440000000000104751762819432769902050495147705078125;-0.0883031999999999983597120944978087209165096282958984375;-0.448761000000000020992985128032159991562366485595703125;0.051278999999999998193889183539795340038836002349853515625]
      mask Display: e = 0.06;c1 = [120 150 230]/255;c2 = ([120 150 230]+0)/383;c3 = ([120 150 230]+128)/383;patch([0 1 1 0],[0 0 1 1],c1);patch([0 1 1 1-e 1-e 0+e],[0 0 1 1-e 0+e 0+e],c2);patch([0 0 1 1-e 0+e 0+e],[0 1 1 1-e 1-e 0+e],c3);plot([0 1 1 0 0],[0 0 1 1 0]);disp('b');
  netsum : Sum  Inputs=2  SaturateOnIntegerOverflow=on  InputSameDT=on  OutDataTypeStr=Inherit: Same as first input
      mask Display: e = 0.06;c1 = [120 150 230]/255;c2 = ([120 150 230]+0)/383;c3 = ([120 150 230]+128)/383;patch([0 1 1 0],[0 0 1 1],c1);patch([0 1 1 1-e 1-e 0+e],[0 0 1 1-e 0+e 0+e],c2);patch([0 0 1 1-e 0+e 0+e],[0 1 1 1-e 1-e 0+e],c3);plot([0 1 1 0 0],[0 0 1 1 0]);disp('+');
  poslin : neural/Transfer Functions/poslin
      settings: SourceProductName=Deep Learning Toolbox  SourceProductBaseCode=NN
  "a{4}" : Outport  InitialOutput=[0;0;0;0;0;0;0;0;0;0;0;0;0;0;0;0;0;0;0;0;0;0;0;0;0;0;0;0;0;0;0;0;0;0;0;0;0;0;0;0;0;0;0;0;0;0;0;0;0;0]
      mask Display: e = 0.06;c1 = [120 230 180]/255;c2 = ([120 230 180]+0)/(256+128);c3 = ([120 230 180]+128)/(256+128);patch([0 1 1 0],[0 0 1 1],c1);patch([0 1 1 1-e 1-e 0+e],[0 0 1 1-e 0+e 0+e],c2);patch([0 0 1 1-e 0+e 0+e],[0 1 1 1-e 1-e 0+e],c3);plot([0 1 1 0 0],[0 0 1 1 0]);
  wires:
  poslin/1 -> "a{4}"/1
  netsum/1 -> poslin/1
  "b{4}"/1 -> netsum/2
  "LW{4,3}"/1("lz{4,3}") -> netsum/1
  Delays 1/1("pd{4,3}") -> "LW{4,3}"/1("ad{4,3}")
  "a{3} "/1 -> Delays 1/1("p{4}")
